simulink model "AcasXuClosedLoop_all_comp"  (Simulink 10.0)
settings: Solver=VariableStepAuto  StartTime=0.0  StopTime=20  RelTol=1e-3
libraries: neural x14040, simulink x2
(part 10 of 534)

== system AcasXuClosedLoop_all_comp/networks/Networks_m_2/AcasXu_1_2/Layer 5 ==
  "a{4} " : Inport  PortDimensions=50
      mask Display: e = 0.06;c1 = [120 230 180]/255;c2 = ([120 230 180]+0)/(256+128);c3 = ([120 230 180]+128)/(256+128);patch([0 1 1 0],[0 0 1 1],c1);patch([0 1 1 1-e 1-e 0+e],[0 0 1 1-e 0+e 0+e],c2);patch([0 0 1 1-e 0+e 0+e],[0 1 1 1-e 1-e 0+e],c3);plot([0 1 1 0 0],[0 0 1 1 0]);
  Delays 1 : Subsystem
      mask Display: e = 0.06;c1 = [120 150 230]/255;c2 = ([120 150 230]+0)/383;c3 = ([120 150 230]+128)/383;patch([0 1 1 0],[0 0 1 1],c1);patch([0 1 1 1-e 1-e 0+e],[0 0 1 1-e 0+e 0+e],c2);patch([0 0 1 1-e 0+e 0+e],[0 1 1 1-e 1-e 0+e],c3);plot([0 1 1 0 0],[0 0 1 1 0]);disp('0');
  "LW{5,4}" : Subsystem
      mask Display: e = 0.06;c1 = [120 150 230]/255;c2 = ([120 150 230]+0)/383;c3 = ([120 150 230]+128)/383;patch([0 1 1 0],[0 0 1 1],c1);patch([0 1 1 1-e 1-e 0+e],[0 0 1 1-e 0+e 0+e],c2);patch([0 0 1 1-e 0+e 0+e],[0 1 1 1-e 1-e 0+e],c3);plot([0 1 1 0 0],[0 0 1 1 0]);disp('W');
  "b{5}" : Constant  Value=[-3.09175000000000022026824808563105762004852294921875;-1.5789699999999999846522769075818359851837158203125;-0.74790400000000001323741116721066646277904510498046875;0.418426000000000020140333845120039768517017364501953125;-0.018132399999999999906652448089516838081181049346923828125;0.7196780000000000399040800402872264385223388671875;0.1770190000000000096758157042131642811000347137451171875;-0.259960000000000024389379404965438880026340484619140625;-2.82767999999999997129407347529195249080657958984375;-0.46265400000000000968469748841016553342342376708984375;-3.398219999999999796358451931155286729335784912109375;1.55956999999999990080823408789001405239105224609375;0.96224100000000001298161578233703039586544036865234375;-2.47121000000000012875034371973015367984771728515625;-0.042217499999999998083755059496979811228811740875244140625;0.29803400000000002112443553414777852594852447509765625;-0.62363299999999999290167806975659914314746856689453125;-0.1538940000000000030144775564622250385582447052001953125;-0.1909220000000000083684170704145799390971660614013671875;-0.435248000000000023756996370138949714601039886474609375;0.45485399999999998055244532224605791270732879638671875;0.197116000000000013425704992187093012034893035888671875;0.02747940000000000104751762819432769902050495147705078125;-1.5378700000000000702726765666739083826541900634765625;1.596940000000000026147972675971686840057373046875;-1.3756299999999999084110413605230860412120819091796875;0.65624899999999997124433548378874547779560089111328125;0.0542021000000000030549784924005507491528987884521484375;-0.6906120000000000036521896618069149553775787353515625;-0.117754999999999998561150960085797123610973358154296875;-0.1625709999999999932907002175852539949119091033935546875;-0.76035200000000002784616981443832628428936004638671875;-0.319209000000000020502710640357690863311290740966796875;-0.465017000000000013670842236024327576160430908203125;0.1303910000000000068975936073911725543439388275146484375;0.0582017999999999979809928163376753218472003936767578125;1.6693700000000000205346850634668953716754913330078125;-0.413046000000000024243718144134618341922760009765625;-0.93940400000000001679012484601116739213466644287109375;-1.9987999999999999101163439263473264873027801513671875;0.44529900000000000037658764995285309851169586181640625;-1.321730000000000071480599217466078698635101318359375;0.44816299999999997805133489237050525844097137451171875;-0.069501800000000002466293835823307745158672332763671875;0.337838000000000027167601501787430606782436370849609375;1.7101599999999999024424823801382444798946380615234375;1.6649700000000000610356210017926059663295745849609375;0.54888499999999995626609461396583355963230133056640625;-0.51785099999999995024069221472018398344516754150390625;0.66518999999999994798827174236066639423370361328125]
      mask Display: e = 0.06;c1 = [120 150 230]/255;c2 = ([120 150 230]+0)/383;c3 = ([120 150 230]+128)/383;patch([0 1 1 0],[0 0 1 1],c1);patch([0 1 1 1-e 1-e 0+e],[0 0 1 1-e 0+e 0+e],c2);patch([0 0 1 1-e 0+e 0+e],[0 1 1 1-e 1-e 0+e],c3);plot([0 1 1 0 0],[0 0 1 1 0]);disp('b');
  netsum : Sum  Inputs=2  SaturateOnIntegerOverflow=on  InputSameDT=on  OutDataTypeStr=Inherit: Same as first input
      mask Display: e = 0.06;c1 = [120 150 230]/255;c2 = ([120 150 230]+0)/383;c3 = ([120 150 230]+128)/383;patch([0 1 1 0],[0 0 1 1],c1);patch([0 1 1 1-e 1-e 0+e],[0 0 1 1-e 0+e 0+e],c2);patch([0 0 1 1-e 0+e 0+e],[0 1 1 1-e 1-e 0+e],c3);plot([0 1 1 0 0],[0 0 1 1 0]);disp('+');
  poslin : neural/Transfer Functions/poslin
      settings: SourceProductName=Deep Learning Toolbox  SourceProductBaseCode=NN
  "a{5}" : Outport  InitialOutput=[0;0;0;0;0;0;0;0;0;0;0;0;0;0;0;0;0;0;0;0;0;0;0;0;0;0;0;0;0;0;0;0;0;0;0;0;0;0;0;0;0;0;0;0;0;0;0;0;0;0]
      mask Display: e = 0.06;c1 = [120 230 180]/255;c2 = ([120 230 180]+0)/(256+128);c3 = ([120 230 180]+128)/(256+128);patch([0 1 1 0],[0 0 1 1],c1);patch([0 1 1 1-e 1-e 0+e],[0 0 1 1-e 0+e 0+e],c2);patch([0 0 1 1-e 0+e 0+e],[0 1 1 1-e 1-e 0+e],c3);plot([0 1 1 0 0],[0 0 1 1 0]);
  wires:
  poslin/1 -> "a{5}"/1
  netsum/1 -> poslin/1
  "b{5}"/1 -> netsum/2
  "LW{5,4}"/1("lz{5,4}") -> netsum/1
  Delays 1/1("pd{5,4}") -> "LW{5,4}"/1("ad{5,4}")
  "a{4} "/1 -> Delays 1/1("p{5}")

== system AcasXuClosedLoop_all_comp/networks/Networks_m_2/AcasXu_1_2/Layer 6 ==
  "a{5} " : Inport  PortDimensions=50
      mask Display: e = 0.06;c1 = [120 230 180]/255;c2 = ([120 230 180]+0)/(256+128);c3 = ([120 230 180]+128)/(256+128);patch([0 1 1 0],[0 0 1 1],c1);patch([0 1 1 1-e 1-e 0+e],[0 0 1 1-e 0+e 0+e],c2);patch([0 0 1 1-e 0+e 0+e],[0 1 1 1-e 1-e 0+e],c3);plot([0 1 1 0 0],[0 0 1 1 0]);
  Delays 1 : Subsystem
      mask Display: e = 0.06;c1 = [120 150 230]/255;c2 = ([120 150 230]+0)/383;c3 = ([120 150 230]+128)/383;patch([0 1 1 0],[0 0 1 1],c1);patch([0 1 1 1-e 1-e 0+e],[0 0 1 1-e 0+e 0+e],c2);patch([0 0 1 1-e 0+e 0+e],[0 1 1 1-e 1-e 0+e],c3);plot([0 1 1 0 0],[0 0 1 1 0]);disp('0');
  "LW{6,5}" : Subsystem
      mask Display: e = 0.06;c1 = [120 150 230]/255;c2 = ([120 150 230]+0)/383;c3 = ([120 150 230]+128)/383;patch([0 1 1 0],[0 0 1 1],c1);patch([0 1 1 1-e 1-e 0+e],[0 0 1 1-e 0+e 0+e],c2);patch([0 0 1 1-e 0+e 0+e],[0 1 1 1-e 1-e 0+e],c3);plot([0 1 1 0 0],[0 0 1 1 0]);disp('W');
  "b{6}" : Constant  Value=[-0.341378000000000014768630762773682363331317901611328125;-1.752289999999999903224079389474354684352874755859375;-0.2081030000000000101945119013180374167859554290771484375;-0.1790799999999999891908686322494759224355220794677734375;-1.8451100000000000278532752417959272861480712890625;0.09219460000000000154951607100883848033845424652099609375;0.19085099999999999287325636032619513571262359619140625;0.1194800000000000028688162956314045004546642303466796875;-2.408889999999999975699438437004573643207550048828125;-4.517640000000000100044417195022106170654296875;-0.15426200000000001022470996758784167468547821044921875;-1.578440000000000065227823142777197062969207763671875;-3.6915800000000000835598257253877818584442138671875;0.91479699999999997128696804793435148894786834716796875;-0.38159799999999999275956952260457910597324371337890625;-1.4166499999999999648281345798750407993793487548828125;0.4794840000000000213020712180878035724163055419921875;0.0837204000000000003733902076419326476752758026123046875;-0.149436999999999986510346161594497971236705780029296875;-0.476080000000000003179678742526448331773281097412109375;-2.065690000000000026147972675971686840057373046875;-0.488171999999999994823696169987670145928859710693359375;-5.1282399999999999096189640113152563571929931640625;-0.326983999999999996877164676334359683096408843994140625;0.029691399999999999737010369926792918704450130462646484375;0.159138000000000001676880856393836438655853271484375;0.169696000000000013496759265763103030622005462646484375;-0.7378299999999999858601995583740063011646270751953125;-0.377545999999999992713384244780172593891620635986328125;0.1332689999999999985735854579615988768637180328369140625;0.1173160000000000036113334545007091946899890899658203125;0.084497400000000000286348722511320374906063079833984375;-5.8619000000000003325340003357268869876861572265625;-1.7486800000000000121502807814977131783962249755859375;1.5344800000000000661515286992653273046016693115234375;-0.42604399999999997827870856781373731791973114013671875;0.1529330000000000133741906438444857485592365264892578125;0.443421000000000009588774219082552008330821990966796875;-0.54626600000000002932409870481933467090129852294921875;-1.263570000000000081996631706715561449527740478515625;0.2061469999999999969109154562829644419252872467041015625;-0.86114699999999999580069243165780790150165557861328125;-0.09318210000000000381437104124415782280266284942626953125;0.23265299999999999869260136620141565799713134765625;0.325915000000000010249578963339445181190967559814453125;1.28146000000000004348521542851813137531280517578125;0.473376999999999992230215184463304467499256134033203125;-0.14630799999999999361222080551669932901859283447265625;0.080574599999999996224886444906587712466716766357421875;-0.1077599999999999946798112659962498582899570465087890625]
      mask Display: e = 0.06;c1 = [120 150 230]/255;c2 = ([120 150 230]+0)/383;c3 = ([120 150 230]+128)/383;patch([0 1 1 0],[0 0 1 1],c1);patch([0 1 1 1-e 1-e 0+e],[0 0 1 1-e 0+e 0+e],c2);patch([0 0 1 1-e 0+e 0+e],[0 1 1 1-e 1-e 0+e],c3);plot([0 1 1 0 0],[0 0 1 1 0]);disp('b');
  netsum : Sum  Inputs=2  SaturateOnIntegerOverflow=on  InputSameDT=on  OutDataTypeStr=Inherit: Same as first input
      mask Display: e = 0.06;c1 = [120 150 230]/255;c2 = ([120 150 230]+0)/383;c3 = ([120 150 230]+128)/383;patch([0 1 1 0],[0 0 1 1],c1);patch([0 1 1 1-e 1-e 0+e],[0 0 1 1-e 0+e 0+e],c2);patch([0 0 1 1-e 0+e 0+e],[0 1 1 1-e 1-e 0+e],c3);plot([0 1 1 0 0],[0 0 1 1 0]);disp('+');
  poslin : neural/Transfer Functions/poslin
      settings: SourceProductName=Deep Learning Toolbox  SourceProductBaseCode=NN
  "a{6}" : Outport  InitialOutput=[0;0;0;0;0;0;0;0;0;0;0;0;0;0;0;0;0;0;0;0;0;0;0;0;0;0;0;0;0;0;0;0;0;0;0;0;0;0;0;0;0;0;0;0;0;0;0;0;0;0]
      mask Display: e = 0.06;c1 = [120 230 180]/255;c2 = ([120 230 180]+0)/(256+128);c3 = ([120 230 180]+128)/(256+128);patch([0 1 1 0],[0 0 1 1],c1);patch([0 1 1 1-e 1-e 0+e],[0 0 1 1-e 0+e 0+e],c2);patch([0 0 1 1-e 0+e 0+e],[0 1 1 1-e 1-e 0+e],c3);plot([0 1 1 0 0],[0 0 1 1 0]);
  wires:
  poslin/1 -> "a{6}"/1
  netsum/1 -> poslin/1
  "b{6}"/1 -> netsum/2
  "LW{6,5}"/1("lz{6,5}") -> netsum/1
  Delays 1/1("pd{6,5}") -> "LW{6,5}"/1("ad{6,5}")
  "a{5} "/1 -> Delays 1/1("p{6}")

== system AcasXuClosedLoop_all_comp/networks/Networks_m_2/AcasXu_1_2/Layer 7 ==
  "a{6} " : Inport  PortDimensions=50
      mask Display: e = 0.06;c1 = [120 230 180]/255;c2 = ([120 230 180]+0)/(256+128);c3 = ([120 230 180]+128)/(256+128);patch([0 1 1 0],[0 0 1 1],c1);patch([0 1 1 1-e 1-e 0+e],[0 0 1 1-e 0+e 0+e],c2);patch([0 0 1 1-e 0+e 0+e],[0 1 1 1-e 1-e 0+e],c3);plot([0 1 1 0 0],[0 0 1 1 0]);
  Delays 1 : Subsystem
      mask Display: e = 0.06;c1 = [120 150 230]/255;c2 = ([120 150 230]+0)/383;c3 = ([120 150 230]+128)/383;patch([0 1 1 0],[0 0 1 1],c1);patch([0 1 1 1-e 1-e 0+e],[0 0 1 1-e 0+e 0+e],c2);patch([0 0 1 1-e 0+e 0+e],[0 1 1 1-e 1-e 0+e],c3);plot([0 1 1 0 0],[0 0 1 1 0]);disp('0');
  "LW{7,6}" : Subsystem
      mask Display: e = 0.06;c1 = [120 150 230]/255;c2 = ([120 150 230]+0)/383;c3 = ([120 150 230]+128)/383;patch([0 1 1 0],[0 0 1 1],c1);patch([0 1 1 1-e 1-e 0+e],[0 0 1 1-e 0+e 0+e],c2);patch([0 0 1 1-e 0+e 0+e],[0 1 1 1-e 1-e 0+e],c3);plot([0 1 1 0 0],[0 0 1 1 0]);disp('W');
  "b{7}" : Constant  Value=[-0.0202845000000000004469757897140880231745541095733642578125;-0.019308499999999999274802320314847747795283794403076171875;-0.019157000000000000416999768049208796583116054534912109375;-0.018912800000000000444355663375972653739154338836669921875;-0.0189197000000000010999645638776200939901173114776611328125]
      mask Display: e = 0.06;c1 = [120 150 230]/255;c2 = ([120 150 230]+0)/383;c3 = ([120 150 230]+128)/383;patch([0 1 1 0],[0 0 1 1],c1);patch([0 1 1 1-e 1-e 0+e],[0 0 1 1-e 0+e 0+e],c2);patch([0 0 1 1-e 0+e 0+e],[0 1 1 1-e 1-e 0+e],c3);plot([0 1 1 0 0],[0 0 1 1 0]);disp('b');
  netsum : Sum  Inputs=2  SaturateOnIntegerOverflow=on  InputSameDT=on  OutDataTypeStr=Inherit: Same as first input
      mask Display: e = 0.06;c1 = [120 150 230]/255;c2 = ([120 150 230]+0)/383;c3 = ([120 150 230]+128)/383;patch([0 1 1 0],[0 0 1 1],c1);patch([0 1 1 1-e 1-e 0+e],[0 0 1 1-e 0+e 0+e],c2);patch([0 0 1 1-e 0+e 0+e],[0 1 1 1-e 1-e 0+e],c3);plot([0 1 1 0 0],[0 0 1 1 0]);disp('+');
  purelin : neural/Transfer Functions/purelin
      settings: SourceProductName=Deep Learning Toolbox
  "a{7}" : Outport  InitialOutput=[0;0;0;0;0]
      mask Display: e = 0.06;c1 = [120 230 180]/255;c2 = ([120 230 180]+0)/(256+128);c3 = ([120 230 180]+128)/(256+128);patch([0 1 1 0],[0 0 1 1],c1);patch([0 1 1 1-e 1-e 0+e],[0 0 1 1-e 0+e 0+e],c2);patch([0 0 1 1-e 0+e 0+e],[0 1 1 1-e 1-e 0+e],c3);plot([0 1 1 0 0],[0 0 1 1 0]);
  wires:
  purelin/1 -> "a{7}"/1
  netsum/1 -> purelin/1
  "b{7}"/1 -> netsum/2
  "LW{7,6}"/1("lz{7,6}") -> netsum/1
  Delays 1/1("pd{7,6}") -> "LW{7,6}"/1("ad{7,6}")
  "a{6} "/1 -> Delays 1/1("p{7}")

== system AcasXuClosedLoop_all_comp/networks/Networks_m_2/AcasXu_1_2/Process Input 1 ==
  x : Inport  PortDimensions=5
      mask Display: e = 0.06;c1 = [120 230 180]/255;c2 = ([120 230 180]+0)/(256+128);c3 = ([120 230 180]+128)/(256+128);patch([0 1 1 0],[0 0 1 1],c1);patch([0 1 1 1-e 1-e 0+e],[0 0 1 1-e 0+e 0+e],c2);patch([0 0 1 1-e 0+e 0+e],[0 1 1 1-e 1-e 0+e],c3);plot([0 1 1 0 0],[0 0 1 1 0]);
  p : Outport  PortDimensions=5
      mask Display: e = 0.06;c1 = [120 230 180]/255;c2 = ([120 230 180]+0)/(256+128);c3 = ([120 230 180]+128)/(256+128);patch([0 1 1 0],[0 0 1 1],c1);patch([0 1 1 1-e 1-e 0+e],[0 0 1 1-e 0+e 0+e],c2);patch([0 0 1 1-e 0+e 0+e],[0 1 1 1-e 1-e 0+e],c3);plot([0 1 1 0 0],[0 0 1 1 0]);
  wires:
  x/1 -> p/1

== system AcasXuClosedLoop_all_comp/networks/Networks_m_2/AcasXu_1_2/Process Output 1 ==
  a : Inport  PortDimensions=5
      mask Display: e = 0.06;c1 = [120 230 180]/255;c2 = ([120 230 180]+0)/(256+128);c3 = ([120 230 180]+128)/(256+128);patch([0 1 1 0],[0 0 1 1],c1);patch([0 1 1 1-e 1-e 0+e],[0 0 1 1-e 0+e 0+e],c2);patch([0 0 1 1-e 0+e 0+e],[0 1 1 1-e 1-e 0+e],c3);plot([0 1 1 0 0],[0 0 1 1 0]);
  y : Outport  PortDimensions=5
      mask Display: e = 0.06;c1 = [120 230 180]/255;c2 = ([120 230 180]+0)/(256+128);c3 = ([120 230 180]+128)/(256+128);patch([0 1 1 0],[0 0 1 1],c1);patch([0 1 1 1-e 1-e 0+e],[0 0 1 1-e 0+e 0+e],c2);patch([0 0 1 1-e 0+e 0+e],[0 1 1 1-e 1-e 0+e],c3);plot([0 1 1 0 0],[0 0 1 1 0]);
  wires:
  a/1 -> y/1

== system AcasXuClosedLoop_all_comp/networks/Networks_m_2/AcasXu_2_2/Layer 1 ==
  "p{1}" : Inport  PortDimensions=5
      mask Display: e = 0.06;c1 = [120 230 180]/255;c2 = ([120 230 180]+0)/(256+128);c3 = ([120 230 180]+128)/(256+128);patch([0 1 1 0],[0 0 1 1],c1);patch([0 1 1 1-e 1-e 0+e],[0 0 1 1-e 0+e 0+e],c2);patch([0 0 1 1-e 0+e 0+e],[0 1 1 1-e 1-e 0+e],c3);plot([0 1 1 0 0],[0 0 1 1 0]);
  Delays 1 : Subsystem
      mask Display: e = 0.06;c1 = [120 150 230]/255;c2 = ([120 150 230]+0)/383;c3 = ([120 150 230]+128)/383;patch([0 1 1 0],[0 0 1 1],c1);patch([0 1 1 1-e 1-e 0+e],[0 0 1 1-e 0+e 0+e],c2);patch([0 0 1 1-e 0+e 0+e],[0 1 1 1-e 1-e 0+e],c3);plot([0 1 1 0 0],[0 0 1 1 0]);disp('0');
  "IW{1,1}" : Subsystem
      mask Display: e = 0.06;c1 = [120 150 230]/255;c2 = ([120 150 230]+0)/383;c3 = ([120 150 230]+128)/383;patch([0 1 1 0],[0 0 1 1],c1);patch([0 1 1 1-e 1-e 0+e],[0 0 1 1-e 0+e 0+e],c2);patch([0 0 1 1-e 0+e 0+e],[0 1 1 1-e 1-e 0+e],c3);plot([0 1 1 0 0],[0 0 1 1 0]);disp('W');
  "b{1}" : Constant  Value=[-0.71073200000000003040412366317468695342540740966796875;-0.023406599999999999628386149197467602789402008056640625;-0.1468069999999999930562211147844209335744380950927734375;0.1924019999999999896989066883179475553333759307861328125;0.34060699999999999310063003576942719519138336181640625;0.0814416000000000028791191652999259531497955322265625;-0.21351500000000001033839680530945770442485809326171875;0.127441999999999999726441046732361428439617156982421875;-0.1905419999999999891571178523008711636066436767578125;-0.1620499999999999996003197111349436454474925994873046875;-0.082190499999999999669597627871553413569927215576171875;-0.64436499999999996557420445242314599454402923583984375;-0.53278400000000003533529024934978224337100982666015625;-0.60548100000000004694555855166981928050518035888671875;-0.0231250999999999991285637435112221282906830310821533203125;-0.0289112000000000014809931059289738186635076999664306640625;-0.3672210000000000196251903616939671337604522705078125;-0.277160000000000017461587731304462067782878875732421875;0.246352999999999988656185223590000532567501068115234375;-0.059020099999999998952038282595822238363325595855712890625;-0.59103399999999994829380511873750947415828704833984375;-0.2083589999999999886615142941082012839615345001220703125;-0.67096599999999995134203345514833927154541015625;0.304474999999999995647925743469386361539363861083984375;-0.1941980000000000095017327339519397355616092681884765625;-0.0832877999999999951707962964064790867269039154052734375;-0.50439299999999998025401737322681583464145660400390625;0.1512320000000000053130833066461491398513317108154296875;0.0917239999999999999769073610877967439591884613037109375;-0.39220500000000002582822844487964175641536712646484375;0.034806999999999997663646666978820576332509517669677734375;-0.0283214000000000000467625937972115934826433658599853515625;0.117306999999999994610533349259640090167522430419921875;-0.45529900000000000925837184695410542190074920654296875;-0.2465559999999999973852027324028313159942626953125;0.11519100000000000172395431263794307596981525421142578125;-0.004594850000000000149469325805284825037233531475067138671875;0.1917580000000000117754694883842603303492069244384765625;-0.765735999999999972231989886495284736156463623046875;-0.056882500000000002504663143554353155195713043212890625;-0.6090430000000000010373923942097462713718414306640625;0.0852509999999999934505723331312765367329120635986328125;-0.3668839999999999879065626373630948364734649658203125;-0.0160115000000000014257484082236260292120277881622314453125;0.1520410000000000094733110245215357281267642974853515625;-0.50538600000000000189714910447946749627590179443359375;0.0280642000000000009507505893679990549571812152862548828125;-0.11279799999999999549071816318246419541537761688232421875;-0.5673850000000000282085466096759773790836334228515625;-0.2479320000000000134843247678873012773692607879638671875]
      mask Display: e = 0.06;c1 = [120 150 230]/255;c2 = ([120 150 230]+0)/383;c3 = ([120 150 230]+128)/383;patch([0 1 1 0],[0 0 1 1],c1);patch([0 1 1 1-e 1-e 0+e],[0 0 1 1-e 0+e 0+e],c2);patch([0 0 1 1-e 0+e 0+e],[0 1 1 1-e 1-e 0+e],c3);plot([0 1 1 0 0],[0 0 1 1 0]);disp('b');
  netsum : Sum  Inputs=2  SaturateOnIntegerOverflow=on  InputSameDT=on  OutDataTypeStr=Inherit: Same as first input
      mask Display: e = 0.06;c1 = [120 150 230]/255;c2 = ([120 150 230]+0)/383;c3 = ([120 150 230]+128)/383;patch([0 1 1 0],[0 0 1 1],c1);patch([0 1 1 1-e 1-e 0+e],[0 0 1 1-e 0+e 0+e],c2);patch([0 0 1 1-e 0+e 0+e],[0 1 1 1-e 1-e 0+e],c3);plot([0 1 1 0 0],[0 0 1 1 0]);disp('+');
  poslin : neural/Transfer Functions/poslin
      settings: SourceProductName=Deep Learning Toolbox  SourceProductBaseCode=NN
  "a{1}" : Outport  InitialOutput=[0;0;0;0;0;0;0;0;0;0;0;0;0;0;0;0;0;0;0;0;0;0;0;0;0;0;0;0;0;0;0;0;0;0;0;0;0;0;0;0;0;0;0;0;0;0;0;0;0;0]
      mask Display: e = 0.06;c1 = [120 230 180]/255;c2 = ([120 230 180]+0)/(256+128);c3 = ([120 230 180]+128)/(256+128);patch([0 1 1 0],[0 0 1 1],c1);patch([0 1 1 1-e 1-e 0+e],[0 0 1 1-e 0+e 0+e],c2);patch([0 0 1 1-e 0+e 0+e],[0 1 1 1-e 1-e 0+e],c3);plot([0 1 1 0 0],[0 0 1 1 0]);
  wires:
  poslin/1 -> "a{1}"/1
  netsum/1 -> poslin/1
  "b{1}"/1 -> netsum/2
  "IW{1,1}"/1("iz{1,1}") -> netsum/1
  Delays 1/1("pd{1,1}") -> "IW{1,1}"/1("pd{1,1}")
  "p{1}"/1 -> Delays 1/1("p{1}")

== system AcasXuClosedLoop_all_comp/networks/Networks_m_2/AcasXu_2_2/Layer 2 ==
  "a{1} " : Inport  PortDimensions=50
      mask Display: e = 0.06;c1 = [120 230 180]/255;c2 = ([120 230 180]+0)/(256+128);c3 = ([120 230 180]+128)/(256+128);patch([0 1 1 0],[0 0 1 1],c1);patch([0 1 1 1-e 1-e 0+e],[0 0 1 1-e 0+e 0+e],c2);patch([0 0 1 1-e 0+e 0+e],[0 1 1 1-e 1-e 0+e],c3);plot([0 1 1 0 0],[0 0 1 1 0]);
  Delays 1 : Subsystem
      mask Display: e = 0.06;c1 = [120 150 230]/255;c2 = ([120 150 230]+0)/383;c3 = ([120 150 230]+128)/383;patch([0 1 1 0],[0 0 1 1],c1);patch([0 1 1 1-e 1-e 0+e],[0 0 1 1-e 0+e 0+e],c2);patch([0 0 1 1-e 0+e 0+e],[0 1 1 1-e 1-e 0+e],c3);plot([0 1 1 0 0],[0 0 1 1 0]);disp('0');
  "LW{2,1}" : Subsystem
      mask Display: e = 0.06;c1 = [120 150 230]/255;c2 = ([120 150 230]+0)/383;c3 = ([120 150 230]+128)/383;patch([0 1 1 0],[0 0 1 1],c1);patch([0 1 1 1-e 1-e 0+e],[0 0 1 1-e 0+e 0+e],c2);patch([0 0 1 1-e 0+e 0+e],[0 1 1 1-e 1-e 0+e],c3);plot([0 1 1 0 0],[0 0 1 1 0]);disp('W');
  "b{2}" : Constant  Value=[-0.12164899999999999324895583185934810899198055267333984375;-0.27929700000000001747224587234086357057094573974609375;-0.09955039999999999722479770980498869903385639190673828125;-0.88822199999999995601029922909219749271869659423828125;0.8940550000000000441247038907022215425968170166015625;-0.85196600000000000108002495835535228252410888671875;-0.1104360000000000063824501239650999195873737335205078125;-0.198512999999999995015542708642897196114063262939453125;-0.0740760000000000029540814239226165227591991424560546875;-1.153350000000000097344354799133725464344024658203125;-0.63325299999999995481658743301522918045520782470703125;-0.94377900000000003455369324001367203891277313232421875;-0.01792950000000000099209529480503988452255725860595703125;0.040906299999999999605915235179054434411227703094482421875;0.170406000000000001914912672873470000922679901123046875;-0.68770399999999998197353079376625828444957733154296875;0.3030559999999999920561322142020799219608306884765625;0.255921000000000009588774219082552008330821990966796875;-0.99250000000000004884981308350688777863979339599609375;0.1765649999999999997246646898929611779749393463134765625;-0.655877999999999961033836370916105806827545166015625;0.646905999999999981042719809920527040958404541015625;1.4067000000000000614619466432486660778522491455078125;-0.339610000000000022968293933445238508284091949462890625;0.641823000000000032372327041230164468288421630859375;1.604179999999999939319650366087444126605987548828125;0.3667730000000000156745727508678101003170013427734375;-0.84923800000000004839506573262042365968227386474609375;-0.7250959999999999627817715008859522640705108642578125;-0.0085157600000000006901235138911943067796528339385986328125;-0.6992819999999999591722144032246433198451995849609375;-0.11682800000000000129052324382428196258842945098876953125;0.52576699999999998436095438592019490897655487060546875;-0.00648930999999999987004617452157617663033306598663330078125;-0.22376099999999998768629438927746377885341644287109375;-0.37841000000000002412292587905540131032466888427734375;0.519700999999999968537167660542763769626617431640625;0.04915540000000000186997084483664366416633129119873046875;-0.042968399999999996874411323233289294876158237457275390625;-0.63150300000000003652900204542675055563449859619140625;-1.0430900000000000726885218682582490146160125732421875;-0.054936400000000003285283156628793221898376941680908203125;-0.0966757000000000032979841080305050127208232879638671875;-0.08870530000000000081517015360077493824064731597900390625;-0.8591100000000000402877731175976805388927459716796875;-0.61526599999999997958610720161232165992259979248046875;-0.031076499999999999956923346644543926231563091278076171875;-0.945826999999999973312014844850637018680572509765625;-0.91236499999999998156141600702540017664432525634765625;0.391450999999999993406163412146270275115966796875]
      mask Display: e = 0.06;c1 = [120 150 230]/255;c2 = ([120 150 230]+0)/383;c3 = ([120 150 230]+128)/383;patch([0 1 1 0],[0 0 1 1],c1);patch([0 1 1 1-e 1-e 0+e],[0 0 1 1-e 0+e 0+e],c2);patch([0 0 1 1-e 0+e 0+e],[0 1 1 1-e 1-e 0+e],c3);plot([0 1 1 0 0],[0 0 1 1 0]);disp('b');
  netsum : Sum  Inputs=2  SaturateOnIntegerOverflow=on  InputSameDT=on  OutDataTypeStr=Inherit: Same as first input
      mask Display: e = 0.06;c1 = [120 150 230]/255;c2 = ([120 150 230]+0)/383;c3 = ([120 150 230]+128)/383;patch([0 1 1 0],[0 0 1 1],c1);patch([0 1 1 1-e 1-e 0+e],[0 0 1 1-e 0+e 0+e],c2);patch([0 0 1 1-e 0+e 0+e],[0 1 1 1-e 1-e 0+e],c3);plot([0 1 1 0 0],[0 0 1 1 0]);disp('+');
  poslin : neural/Transfer Functions/poslin
      settings: SourceProductName=Deep Learning Toolbox  SourceProductBaseCode=NN
  "a{2}" : Outport  InitialOutput=[0;0;0;0;0;0;0;0;0;0;0;0;0;0;0;0;0;0;0;0;0;0;0;0;0;0;0;0;0;0;0;0;0;0;0;0;0;0;0;0;0;0;0;0;0;0;0;0;0;0]
      mask Display: e = 0.06;c1 = [120 230 180]/255;c2 = ([120 230 180]+0)/(256+128);c3 = ([120 230 180]+128)/(256+128);patch([0 1 1 0],[0 0 1 1],c1);patch([0 1 1 1-e 1-e 0+e],[0 0 1 1-e 0+e 0+e],c2);patch([0 0 1 1-e 0+e 0+e],[0 1 1 1-e 1-e 0+e],c3);plot([0 1 1 0 0],[0 0 1 1 0]);
  wires:
  poslin/1 -> "a{2}"/1
  netsum/1 -> poslin/1
  "b{2}"/1 -> netsum/2
  "LW{2,1}"/1("lz{2,1}") -> netsum/1
  Delays 1/1("pd{2,1}") -> "LW{2,1}"/1("ad{2,1}")
  "a{1} "/1 -> Delays 1/1("p{2}")

== system AcasXuClosedLoop_all_comp/networks/Networks_m_2/AcasXu_2_2/Layer 3 ==
  "a{2} " : Inport  PortDimensions=50
      mask Display: e = 0.06;c1 = [120 230 180]/255;c2 = ([120 230 180]+0)/(256+128);c3 = ([120 230 180]+128)/(256+128);patch([0 1 1 0],[0 0 1 1],c1);patch([0 1 1 1-e 1-e 0+e],[0 0 1 1-e 0+e 0+e],c2);patch([0 0 1 1-e 0+e 0+e],[0 1 1 1-e 1-e 0+e],c3);plot([0 1 1 0 0],[0 0 1 1 0]);
  Delays 1 : Subsystem
      mask Display: e = 0.06;c1 = [120 150 230]/255;c2 = ([120 150 230]+0)/383;c3 = ([120 150 230]+128)/383;patch([0 1 1 0],[0 0 1 1],c1);patch([0 1 1 1-e 1-e 0+e],[0 0 1 1-e 0+e 0+e],c2);patch([0 0 1 1-e 0+e 0+e],[0 1 1 1-e 1-e 0+e],c3);plot([0 1 1 0 0],[0 0 1 1 0]);disp('0');
  "LW{3,2}" : Subsystem
      mask Display: e = 0.06;c1 = [120 150 230]/255;c2 = ([120 150 230]+0)/383;c3 = ([120 150 230]+128)/383;patch([0 1 1 0],[0 0 1 1],c1);patch([0 1 1 1-e 1-e 0+e],[0 0 1 1-e 0+e 0+e],c2);patch([0 0 1 1-e 0+e 0+e],[0 1 1 1-e 1-e 0+e],c3);plot([0 1 1 0 0],[0 0 1 1 0]);disp('W');
  "b{3}" : Constant  Value=[-0.264120999999999994667376768120448105037212371826171875;1.019439999999999901802993917954154312610626220703125;1.366330000000000044479975258582271635532379150390625;0.400241999999999986670218277140520513057708740234375;0.920061000000000017706724975141696631908416748046875;2.51973000000000002529532139305956661701202392578125;-0.1667039999999999910773595956925419159233570098876953125;-1.8284599999999999742072986919083632528781890869140625;0.56852300000000000057553961596568115055561065673828125;0.195977000000000012303047469686134718358516693115234375;-0.0461897000000000002017941369558684527873992919921875;0.063801099999999999479172174687846563756465911865234375;-0.36269899999999999362643166023190133273601531982421875;-0.45260299999999997755395497733843512833118438720703125;0.7419350000000000111555209514335729181766510009765625;-0.78901100000000001788436065908172167837619781494140625;-0.11247400000000000452882176205093855969607830047607421875;-0.0411870000000000013873346915715956129133701324462890625;0.424572000000000004948930154569097794592380523681640625;-0.1323910000000000086739504467914230190217494964599609375;-0.70211999999999996635580146175925619900226593017578125;-0.155287000000000008359535286217578686773777008056640625;0.00733787999999999974998221574651324772275984287261962890625;-0.58643599999999995731059243553318083286285400390625;-0.07315449999999999730615485304952017031610012054443359375;0.472536000000000011578293879210832528769969940185546875;-1.15599000000000007304379323613829910755157470703125;-0.606435999999999975074160829535685479640960693359375;1.2991299999999998959765434847213327884674072265625;0.80531900000000000705568936609779484570026397705078125;2.35156000000000009464429240324534475803375244140625;0.82914399999999999213429191513569094240665435791015625;-0.48423300000000002452082981108105741441249847412109375;-0.90704399999999996140331859351135790348052978515625;-0.040279500000000002801758824944045045413076877593994140625;0.049025300000000000821831491748525877483189105987548828125;-0.32336500000000001353583911622990854084491729736328125;0.198807000000000011485923323562019504606723785400390625;0.01143079999999999964155339426952195935882627964019775390625;-1.39806000000000008043343768804334104061126708984375;-1.37755999999999989569232639041729271411895751953125;-0.27245200000000002749089844655827619135379791259765625;-0.574049000000000031462832339457236230373382568359375;-1.0890999999999999570121644865139387547969818115234375;0.350509000000000014996004438216914422810077667236328125;-1.3330200000000000937205868467572145164012908935546875;1.199829999999999952109419609769247472286224365234375;-1.9733899999999999774757952764048241078853607177734375;1.0203800000000000647304432277451269328594207763671875;1.902930000000000010373923942097462713718414306640625]
      mask Display: e = 0.06;c1 = [120 150 230]/255;c2 = ([120 150 230]+0)/383;c3 = ([120 150 230]+128)/383;patch([0 1 1 0],[0 0 1 1],c1);patch([0 1 1 1-e 1-e 0+e],[0 0 1 1-e 0+e 0+e],c2);patch([0 0 1 1-e 0+e 0+e],[0 1 1 1-e 1-e 0+e],c3);plot([0 1 1 0 0],[0 0 1 1 0]);disp('b');
  netsum : Sum  Inputs=2  SaturateOnIntegerOverflow=on  InputSameDT=on  OutDataTypeStr=Inherit: Same as first input
      mask Display: e = 0.06;c1 = [120 150 230]/255;c2 = ([120 150 230]+0)/383;c3 = ([120 150 230]+128)/383;patch([0 1 1 0],[0 0 1 1],c1);patch([0 1 1 1-e 1-e 0+e],[0 0 1 1-e 0+e 0+e],c2);patch([0 0 1 1-e 0+e 0+e],[0 1 1 1-e 1-e 0+e],c3);plot([0 1 1 0 0],[0 0 1 1 0]);disp('+');
  poslin : neural/Transfer Functions/poslin
      settings: SourceProductName=Deep Learning Toolbox  SourceProductBaseCode=NN
  "a{3}" : Outport  InitialOutput=[0;0;0;0;0;0;0;0;0;0;0;0;0;0;0;0;0;0;0;0;0;0;0;0;0;0;0;0;0;0;0;0;0;0;0;0;0;0;0;0;0;0;0;0;0;0;0;0;0;0]
      mask Display: e = 0.06;c1 = [120 230 180]/255;c2 = ([120 230 180]+0)/(256+128);c3 = ([120 230 180]+128)/(256+128);patch([0 1 1 0],[0 0 1 1],c1);patch([0 1 1 1-e 1-e 0+e],[0 0 1 1-e 0+e 0+e],c2);patch([0 0 1 1-e 0+e 0+e],[0 1 1 1-e 1-e 0+e],c3);plot([0 1 1 0 0],[0 0 1 1 0]);
  wires:
  poslin/1 -> "a{3}"/1
  netsum/1 -> poslin/1
  "b{3}"/1 -> netsum/2
  "LW{3,2}"/1("lz{3,2}") -> netsum/1
  Delays 1/1("pd{3,2}") -> "LW{3,2}"/1("ad{3,2}")
  "a{2} "/1 -> Delays 1/1("p{3}")

== system AcasXuClosedLoop_all_comp/networks/Networks_m_2/AcasXu_2_2/Layer 4 ==
  "a{3} " : Inport  PortDimensions=50
      mask Display: e = 0.06;c1 = [120 230 180]/255;c2 = ([120 230 180]+0)/(256+128);c3 = ([120 230 180]+128)/(256+128);patch([0 1 1 0],[0 0 1 1],c1);patch([0 1 1 1-e 1-e 0+e],[0 0 1 1-e 0+e 0+e],c2);patch([0 0 1 1-e 0+e 0+e],[0 1 1 1-e 1-e 0+e],c3);plot([0 1 1 0 0],[0 0 1 1 0]);
  Delays 1 : Subsystem
      mask Display: e = 0.06;c1 = [120 150 230]/255;c2 = ([120 150 230]+0)/383;c3 = ([120 150 230]+128)/383;patch([0 1 1 0],[0 0 1 1],c1);patch([0 1 1 1-e 1-e 0+e],[0 0 1 1-e 0+e 0+e],c2);patch([0 0 1 1-e 0+e 0+e],[0 1 1 1-e 1-e 0+e],c3);plot([0 1 1 0 0],[0 0 1 1 0]);disp('0');
  "LW{4,3}" : Subsystem
      mask Display: e = 0.06;c1 = [120 150 230]/255;c2 = ([120 150 230]+0)/383;c3 = ([120 150 230]+128)/383;patch([0 1 1 0],[0 0 1 1],c1);patch([0 1 1 1-e 1-e 0+e],[0 0 1 1-e 0+e 0+e],c2);patch([0 0 1 1-e 0+e 0+e],[0 1 1 1-e 1-e 0+e],c3);plot([0 1 1 0 0],[0 0 1 1 0]);disp('W');
  "b{4}" : Constant  Value=[-0.44458100000000000395772303818375803530216217041015625;-0.6136500000000000287769807982840575277805328369140625;1.539800000000000057553961596568115055561065673828125;0.03728309999999999957420726559575996361672878265380859375;1.015309999999999934772176857222802937030792236328125;0.5130829999999999557758201262913644313812255859375;-0.388458000000000025497826072751195169985294342041015625;0.7490010000000000278674860965111292898654937744140625;-0.83132099999999997663735484820790588855743408203125;1.306810000000000027142732506035827100276947021484375;-0.71396599999999998953370550225372426211833953857421875;-1.0359100000000001084998757505672983825206756591796875;0.6877590000000000092228447101661004126071929931640625;-1.599320000000000074891204349114559590816497802734375;-1.0105299999999999283062379618058912456035614013671875;-0.00995919999999999965012431601962816785089671611785888671875;0.64485800000000004228439820508356206119060516357421875;-0.824443000000000036919800550094805657863616943359375;-0.35442499999999999005240169935859739780426025390625;1.27400000000000002131628207280300557613372802734375;-0.26172899999999998943422951924731023609638214111328125;0.1671620000000000050288662123421090655028820037841796875;-0.0224095999999999982710274792907512164674699306488037109375;-0.64144400000000001416111672369879670441150665283203125;0.498035999999999978715692350306198932230472564697265625;-2.885639999999999982804865794605575501918792724609375;-0.60279599999999999848654397283098660409450531005859375;0.8584739999999999593427446598070673644542694091796875;-2.10681999999999991501908880309201776981353759765625;0.86424500000000004096278871656977571547031402587890625;0.631205000000000016058265828178264200687408447265625;1.1473800000000000665068000671453773975372314453125;0.88716799999999995662847140920348465442657470703125;-0.297086000000000016729728713471558876335620880126953125;-0.15964400000000000812150346973794512450695037841796875;-0.1458419999999999994155785998373175971210002899169921875;0.2073649999999999937738692779021221213042736053466796875;-1.4773099999999999010213969086180441081523895263671875;1.3907199999999999562305674771778285503387451171875;-1.79671000000000002927436071331612765789031982421875;-1.353730000000000099902308647870086133480072021484375;0.97067000000000003279154725532862357795238494873046875;0.39004600000000000381561449103173799812793731689453125;-1.051250000000000017763568394002504646778106689453125;0.52064100000000002044231450781808234751224517822265625;-0.4126469999999999860307298149564303457736968994140625;1.4720299999999999496225200346088968217372894287109375;-0.187323999999999990517807191281463019549846649169921875;-0.853366999999999986670218277140520513057708740234375;-1.163699999999999956656893118633888661861419677734375]
      mask Display: e = 0.06;c1 = [120 150 230]/255;c2 = ([120 150 230]+0)/383;c3 = ([120 150 230]+128)/383;patch([0 1 1 0],[0 0 1 1],c1);patch([0 1 1 1-e 1-e 0+e],[0 0 1 1-e 0+e 0+e],c2);patch([0 0 1 1-e 0+e 0+e],[0 1 1 1-e 1-e 0+e],c3);plot([0 1 1 0 0],[0 0 1 1 0]);disp('b');
  netsum : Sum  Inputs=2  SaturateOnIntegerOverflow=on  InputSameDT=on  OutDataTypeStr=Inherit: Same as first input
      mask Display: e = 0.06;c1 = [120 150 230]/255;c2 = ([120 150 230]+0)/383;c3 = ([120 150 230]+128)/383;patch([0 1 1 0],[0 0 1 1],c1);patch([0 1 1 1-e 1-e 0+e],[0 0 1 1-e 0+e 0+e],c2);patch([0 0 1 1-e 0+e 0+e],[0 1 1 1-e 1-e 0+e],c3);plot([0 1 1 0 0],[0 0 1 1 0]);disp('+');
  poslin : neural/Transfer Functions/poslin
      settings: SourceProductName=Deep Learning Toolbox  SourceProductBaseCode=NN
  "a{4}" : Outport  InitialOutput=[0;0;0;0;0;0;0;0;0;0;0;0;0;0;0;0;0;0;0;0;0;0;0;0;0;0;0;0;0;0;0;0;0;0;0;0;0;0;0;0;0;0;0;0;0;0;0;0;0;0]
      mask Display: e = 0.06;c1 = [120 230 180]/255;c2 = ([120 230 180]+0)/(256+128);c3 = ([120 230 180]+128)/(256+128);patch([0 1 1 0],[0 0 1 1],c1);patch([0 1 1 1-e 1-e 0+e],[0 0 1 1-e 0+e 0+e],c2);patch([0 0 1 1-e 0+e 0+e],[0 1 1 1-e 1-e 0+e],c3);plot([0 1 1 0 0],[0 0 1 1 0]);
  wires:
  poslin/1 -> "a{4}"/1
  netsum/1 -> poslin/1
  "b{4}"/1 -> netsum/2
  "LW{4,3}"/1("lz{4,3}") -> netsum/1
  Delays 1/1("pd{4,3}") -> "LW{4,3}"/1("ad{4,3}")
  "a{3} "/1 -> Delays 1/1("p{4}")

== system AcasXuClosedLoop_all_comp/networks/Networks_m_2/AcasXu_2_2/Layer 5 ==
  "a{4} " : Inport  PortDimensions=50
      mask Display: e = 0.06;c1 = [120 230 180]/255;c2 = ([120 230 180]+0)/(256+128);c3 = ([120 230 180]+128)/(256+128);patch([0 1 1 0],[0 0 1 1],c1);patch([0 1 1 1-e 1-e 0+e],[0 0 1 1-e 0+e 0+e],c2);patch([0 0 1 1-e 0+e 0+e],[0 1 1 1-e 1-e 0+e],c3);plot([0 1 1 0 0],[0 0 1 1 0]);
  Delays 1 : Subsystem
      mask Display: e = 0.06;c1 = [120 150 230]/255;c2 = ([120 150 230]+0)/383;c3 = ([120 150 230]+128)/383;patch([0 1 1 0],[0 0 1 1],c1);patch([0 1 1 1-e 1-e 0+e],[0 0 1 1-e 0+e 0+e],c2);patch([0 0 1 1-e 0+e 0+e],[0 1 1 1-e 1-e 0+e],c3);plot([0 1 1 0 0],[0 0 1 1 0]);disp('0');
  "LW{5,4}" : Subsystem
      mask Display: e = 0.06;c1 = [120 150 230]/255;c2 = ([120 150 230]+0)/383;c3 = ([120 150 230]+128)/383;patch([0 1 1 0],[0 0 1 1],c1);patch([0 1 1 1-e 1-e 0+e],[0 0 1 1-e 0+e 0+e],c2);patch([0 0 1 1-e 0+e 0+e],[0 1 1 1-e 1-e 0+e],c3);plot([0 1 1 0 0],[0 0 1 1 0]);disp('W');
  "b{5}" : Constant  Value=[-0.499987000000000014754419908058480359613895416259765625;0.310634999999999994457766661071218550205230712890625;-0.250103000000000019742429913094383664429187774658203125;-0.2603079999999999838422581888153217732906341552734375;-1.77672000000000007702283255639486014842987060546875;-1.648660000000000014352963262354023754596710205078125;-0.352605000000000001758593271006247960031032562255859375;0.736261999999999972033037920482456684112548828125;-0.94427300000000002899724904636968858540058135986328125;-0.31502799999999997471178403429803438484668731689453125;0.435279000000000027004176672562607564032077789306640625;-1.35583000000000009066525308298878371715545654296875;-0.046693900000000003458477948470317642204463481903076171875;-0.96484199999999997743316271225921809673309326171875;0.64108200000000004070699333169613964855670928955078125;-0.557584999999999997299937604111619293689727783203125;-0.83259000000000005226041821515536867082118988037109375;0.391069999999999973194775293450220488011837005615234375;-0.0337700999999999973422148968893452547490596771240234375;-0.325504000000000015546675058430992066860198974609375;-0.0749506000000000061067595424901810474693775177001953125;-0.6806419999999999692619212510180659592151641845703125;0.50772899999999998588151584044680930674076080322265625;1.343669999999999919992887953412719070911407470703125;-0.07546730000000000104787289956220774911344051361083984375;-0.7545960000000000444941861132974736392498016357421875;0.23977999999999999314326259991503320634365081787109375;-0.1722519999999999884554569007377722300589084625244140625;1.8189100000000000267874611381557770073413848876953125;0.55622300000000002295763579240883700549602508544921875;-0.1694199999999999872724032456972054205834865570068359375;-0.1377400000000000013233858453531865961849689483642578125;0.2651310000000000055564441936439834535121917724609375;-0.971028000000000002245315045001916587352752685546875;0.60082599999999997120170291964313946664333343505859375;-1.61577999999999999403144101961515843868255615234375;-1.306880000000000041637804315541870892047882080078125;1.2227399999999999380406734417192637920379638671875;0.371184000000000013930190334576764144003391265869140625;-1.0368500000000000493827201353269629180431365966796875;0.319371000000000015983658840923453681170940399169921875;0.07291499999999999370725589642461272887885570526123046875;-0.85352200000000000290611978925880976021289825439453125;-0.45541500000000001424638185199000872671604156494140625;-2.29389999999999982804865794605575501918792724609375;-0.709149000000000029331204132176935672760009765625;-1.0632800000000000029132252166164107620716094970703125;-0.368613000000000023970159190866979770362377166748046875;-0.032256600000000003325428821199238882400095462799072265625;-1.191920000000000090523144535836763679981231689453125]
      mask Display: e = 0.06;c1 = [120 150 230]/255;c2 = ([120 150 230]+0)/383;c3 = ([120 150 230]+128)/383;patch([0 1 1 0],[0 0 1 1],c1);patch([0 1 1 1-e 1-e 0+e],[0 0 1 1-e 0+e 0+e],c2);patch([0 0 1 1-e 0+e 0+e],[0 1 1 1-e 1-e 0+e],c3);plot([0 1 1 0 0],[0 0 1 1 0]);disp('b');
  netsum : Sum  Inputs=2  SaturateOnIntegerOverflow=on  InputSameDT=on  OutDataTypeStr=Inherit: Same as first input
      mask Display: e = 0.06;c1 = [120 150 230]/255;c2 = ([120 150 230]+0)/383;c3 = ([120 150 230]+128)/383;patch([0 1 1 0],[0 0 1 1],c1);patch([0 1 1 1-e 1-e 0+e],[0 0 1 1-e 0+e 0+e],c2);patch([0 0 1 1-e 0+e 0+e],[0 1 1 1-e 1-e 0+e],c3);plot([0 1 1 0 0],[0 0 1 1 0]);disp('+');
  poslin : neural/Transfer Functions/poslin
      settings: SourceProductName=Deep Learning Toolbox  SourceProductBaseCode=NN
  "a{5}" : Outport  InitialOutput=[0;0;0;0;0;0;0;0;0;0;0;0;0;0;0;0;0;0;0;0;0;0;0;0;0;0;0;0;0;0;0;0;0;0;0;0;0;0;0;0;0;0;0;0;0;0;0;0;0;0]
      mask Display: e = 0.06;c1 = [120 230 180]/255;c2 = ([120 230 180]+0)/(256+128);c3 = ([120 230 180]+128)/(256+128);patch([0 1 1 0],[0 0 1 1],c1);patch([0 1 1 1-e 1-e 0+e],[0 0 1 1-e 0+e 0+e],c2);patch([0 0 1 1-e 0+e 0+e],[0 1 1 1-e 1-e 0+e],c3);plot([0 1 1 0 0],[0 0 1 1 0]);
  wires:
  poslin/1 -> "a{5}"/1
  netsum/1 -> poslin/1
  "b{5}"/1 -> netsum/2
  "LW{5,4}"/1("lz{5,4}") -> netsum/1
  Delays 1/1("pd{5,4}") -> "LW{5,4}"/1("ad{5,4}")
  "a{4} "/1 -> Delays 1/1("p{5}")

== system AcasXuClosedLoop_all_comp/networks/Networks_m_2/AcasXu_2_2/Layer 6 ==
  "a{5} " : Inport  PortDimensions=50
      mask Display: e = 0.06;c1 = [120 230 180]/255;c2 = ([120 230 180]+0)/(256+128);c3 = ([120 230 180]+128)/(256+128);patch([0 1 1 0],[0 0 1 1],c1);patch([0 1 1 1-e 1-e 0+e],[0 0 1 1-e 0+e 0+e],c2);patch([0 0 1 1-e 0+e 0+e],[0 1 1 1-e 1-e 0+e],c3);plot([0 1 1 0 0],[0 0 1 1 0]);
  Delays 1 : Subsystem
      mask Display: e = 0.06;c1 = [120 150 230]/255;c2 = ([120 150 230]+0)/383;c3 = ([120 150 230]+128)/383;patch([0 1 1 0],[0 0 1 1],c1);patch([0 1 1 1-e 1-e 0+e],[0 0 1 1-e 0+e 0+e],c2);patch([0 0 1 1-e 0+e 0+e],[0 1 1 1-e 1-e 0+e],c3);plot([0 1 1 0 0],[0 0 1 1 0]);disp('0');
  "LW{6,5}" : Subsystem
      mask Display: e = 0.06;c1 = [120 150 230]/255;c2 = ([120 150 230]+0)/383;c3 = ([120 150 230]+128)/383;patch([0 1 1 0],[0 0 1 1],c1);patch([0 1 1 1-e 1-e 0+e],[0 0 1 1-e 0+e 0+e],c2);patch([0 0 1 1-e 0+e 0+e],[0 1 1 1-e 1-e 0+e],c3);plot([0 1 1 0 0],[0 0 1 1 0]);disp('W');
  "b{6}" : Constant  Value=[0.2200249999999999983568699235547683201730251312255859375;-0.0478951999999999988855137189602828584611415863037109375;-0.58017700000000005378097966968198306858539581298828125;-0.17723400000000000265032440438517369329929351806640625;-0.57339099999999998402699930011294782161712646484375;-0.364536000000000026677327014112961478531360626220703125;-0.3008830000000000115534248834592290222644805908203125;-1.03763000000000005229594535194337368011474609375;-0.1310099999999999875743839083952479995787143707275390625;-0.3327240000000000197388771994155831634998321533203125;-0.1416469999999999951345586168827139772474765777587890625;-0.1054110000000000046949111265348619781434535980224609375;-0.361391999999999991022292533671134151518344879150390625;-1.0937099999999999599964439767063595354557037353515625;-0.0300049000000000010146550266654230654239654541015625;-0.1162360000000000059827698351000435650348663330078125;-0.050227399999999998436717163485809578560292720794677734375;0.07423839999999999605950762315842439420521259307861328125;-0.4079450000000000020605739337042905390262603759765625;-0.265705000000000024495960815329453907907009124755859375;0.038778600000000003344968746432641637511551380157470703125;-1.0891900000000001025313167701824568212032318115234375;-0.26776400000000000201083594220108352601528167724609375;-1.13712999999999997413624441833235323429107666015625;-0.4884720000000000172946101884008385241031646728515625;0.238605000000000011528555887707625515758991241455078125;0.04211410000000000153352885945423622615635395050048828125;-0.1233910000000000006803446694902959279716014862060546875;-0.531360999999999972231989886495284736156463623046875;-0.1518400000000000027444713168733869679272174835205078125;-0.0726108000000000031182167958831996656954288482666015625;0.13446700000000000319033688356284983456134796142578125;0.04289479999999999682014362178961164318025112152099609375;0.1253640000000000032098768087962525896728038787841796875;0.16803000000000001268318783331778831779956817626953125;-0.544667000000000012249756764504127204418182373046875;-0.4016640000000000210178541237837634980678558349609375;0.08711829999999999574189502027365961112082004547119140625;-0.0340924999999999978061993033406906761229038238525390625;0.15007999999999999118927007657475769519805908203125;0.213195999999999996621369291460723616182804107666015625;0.2033409999999999939745976007543504238128662109375;-0.280411000000000021348256495912210084497928619384765625;-0.12446400000000000518607379262903123162686824798583984375;-0.06340120000000000477502481999181327410042285919189453125;-0.9868230000000000057269744502264074981212615966796875;-0.3010990000000000055280224842135794460773468017578125;-0.1292310000000000125286447882899665273725986480712890625;-0.53115400000000001501376800661091692745685577392578125;-0.022138299999999999645439174855710007250308990478515625]
      mask Display: e = 0.06;c1 = [120 150 230]/255;c2 = ([120 150 230]+0)/383;c3 = ([120 150 230]+128)/383;patch([0 1 1 0],[0 0 1 1],c1);patch([0 1 1 1-e 1-e 0+e],[0 0 1 1-e 0+e 0+e],c2);patch([0 0 1 1-e 0+e 0+e],[0 1 1 1-e 1-e 0+e],c3);plot([0 1 1 0 0],[0 0 1 1 0]);disp('b');
  netsum : Sum  Inputs=2  SaturateOnIntegerOverflow=on  InputSameDT=on  OutDataTypeStr=Inherit: Same as first input
      mask Display: e = 0.06;c1 = [120 150 230]/255;c2 = ([120 150 230]+0)/383;c3 = ([120 150 230]+128)/383;patch([0 1 1 0],[0 0 1 1],c1);patch([0 1 1 1-e 1-e 0+e],[0 0 1 1-e 0+e 0+e],c2);patch([0 0 1 1-e 0+e 0+e],[0 1 1 1-e 1-e 0+e],c3);plot([0 1 1 0 0],[0 0 1 1 0]);disp('+');
  poslin : neural/Transfer Functions/poslin
      settings: SourceProductName=Deep Learning Toolbox  SourceProductBaseCode=NN
  "a{6}" : Outport  InitialOutput=[0;0;0;0;0;0;0;0;0;0;0;0;0;0;0;0;0;0;0;0;0;0;0;0;0;0;0;0;0;0;0;0;0;0;0;0;0;0;0;0;0;0;0;0;0;0;0;0;0;0]
      mask Display: e = 0.06;c1 = [120 230 180]/255;c2 = ([120 230 180]+0)/(256+128);c3 = ([120 230 180]+128)/(256+128);patch([0 1 1 0],[0 0 1 1],c1);patch([0 1 1 1-e 1-e 0+e],[0 0 1 1-e 0+e 0+e],c2);patch([0 0 1 1-e 0+e 0+e],[0 1 1 1-e 1-e 0+e],c3);plot([0 1 1 0 0],[0 0 1 1 0]);
  wires:
  poslin/1 -> "a{6}"/1
  netsum/1 -> poslin/1
  "b{6}"/1 -> netsum/2
  "LW{6,5}"/1("lz{6,5}") -> netsum/1
  Delays 1/1("pd{6,5}") -> "LW{6,5}"/1("ad{6,5}")
  "a{5} "/1 -> Delays 1/1("p{6}")

== system AcasXuClosedLoop_all_comp/networks/Networks_m_2/AcasXu_2_2/Layer 7 ==
  "a{6} " : Inport  PortDimensions=50
      mask Display: e = 0.06;c1 = [120 230 180]/255;c2 = ([120 230 180]+0)/(256+128);c3 = ([120 230 180]+128)/(256+128);patch([0 1 1 0],[0 0 1 1],c1);patch([0 1 1 1-e 1-e 0+e],[0 0 1 1-e 0+e 0+e],c2);patch([0 0 1 1-e 0+e 0+e],[0 1 1 1-e 1-e 0+e],c3);plot([0 1 1 0 0],[0 0 1 1 0]);
  Delays 1 : Subsystem
      mask Display: e = 0.06;c1 = [120 150 230]/255;c2 = ([120 150 230]+0)/383;c3 = ([120 150 230]+128)/383;patch([0 1 1 0],[0 0 1 1],c1);patch([0 1 1 1-e 1-e 0+e],[0 0 1 1-e 0+e 0+e],c2);patch([0 0 1 1-e 0+e 0+e],[0 1 1 1-e 1-e 0+e],c3);plot([0 1 1 0 0],[0 0 1 1 0]);disp('0');
  "LW{7,6}" : Subsystem
      mask Display: e = 0.06;c1 = [120 150 230]/255;c2 = ([120 150 230]+0)/383;c3 = ([120 150 230]+128)/383;patch([0 1 1 0],[0 0 1 1],c1);patch([0 1 1 1-e 1-e 0+e],[0 0 1 1-e 0+e 0+e],c2);patch([0 0 1 1-e 0+e 0+e],[0 1 1 1-e 1-e 0+e],c3);plot([0 1 1 0 0],[0 0 1 1 0]);disp('W');
  "b{7}" : Constant  Value=[-0.0210871000000000009821921054253834881819784641265869140625;-0.0190332000000000001183053655040566809475421905517578125;0.018937900000000000566569013926709885708987712860107421875;-0.0184082999999999989693133528589896741323173046112060546875;0.018053500000000000103028696685214526951313018798828125]
      mask Display: e = 0.06;c1 = [120 150 230]/255;c2 = ([120 150 230]+0)/383;c3 = ([120 150 230]+128)/383;patch([0 1 1 0],[0 0 1 1],c1);patch([0 1 1 1-e 1-e 0+e],[0 0 1 1-e 0+e 0+e],c2);patch([0 0 1 1-e 0+e 0+e],[0 1 1 1-e 1-e 0+e],c3);plot([0 1 1 0 0],[0 0 1 1 0]);disp('b');
  netsum : Sum  Inputs=2  SaturateOnIntegerOverflow=on  InputSameDT=on  OutDataTypeStr=Inherit: Same as first input
      mask Display: e = 0.06;c1 = [120 150 230]/255;c2 = ([120 150 230]+0)/383;c3 = ([120 150 230]+128)/383;patch([0 1 1 0],[0 0 1 1],c1);patch([0 1 1 1-e 1-e 0+e],[0 0 1 1-e 0+e 0+e],c2);patch([0 0 1 1-e 0+e 0+e],[0 1 1 1-e 1-e 0+e],c3);plot([0 1 1 0 0],[0 0 1 1 0]);disp('+');
  purelin : neural/Transfer Functions/purelin
      settings: SourceProductName=Deep Learning Toolbox
  "a{7}" : Outport  InitialOutput=[0;0;0;0;0]
      mask Display: e = 0.06;c1 = [120 230 180]/255;c2 = ([120 230 180]+0)/(256+128);c3 = ([120 230 180]+128)/(256+128);patch([0 1 1 0],[0 0 1 1],c1);patch([0 1 1 1-e 1-e 0+e],[0 0 1 1-e 0+e 0+e],c2);patch([0 0 1 1-e 0+e 0+e],[0 1 1 1-e 1-e 0+e],c3);plot([0 1 1 0 0],[0 0 1 1 0]);
  wires:
  purelin/1 -> "a{7}"/1
  netsum/1 -> purelin/1
  "b{7}"/1 -> netsum/2
  "LW{7,6}"/1("lz{7,6}") -> netsum/1
  Delays 1/1("pd{7,6}") -> "LW{7,6}"/1("ad{7,6}")
  "a{6} "/1 -> Delays 1/1("p{7}")

== system AcasXuClosedLoop_all_comp/networks/Networks_m_2/AcasXu_2_2/Process Input 1 ==
  x : Inport  PortDimensions=5
      mask Display: e = 0.06;c1 = [120 230 180]/255;c2 = ([120 230 180]+0)/(256+128);c3 = ([120 230 180]+128)/(256+128);patch([0 1 1 0],[0 0 1 1],c1);patch([0 1 1 1-e 1-e 0+e],[0 0 1 1-e 0+e 0+e],c2);patch([0 0 1 1-e 0+e 0+e],[0 1 1 1-e 1-e 0+e],c3);plot([0 1 1 0 0],[0 0 1 1 0]);
  p : Outport  PortDimensions=5
      mask Display: e = 0.06;c1 = [120 230 180]/255;c2 = ([120 230 180]+0)/(256+128);c3 = ([120 230 180]+128)/(256+128);patch([0 1 1 0],[0 0 1 1],c1);patch([0 1 1 1-e 1-e 0+e],[0 0 1 1-e 0+e 0+e],c2);patch([0 0 1 1-e 0+e 0+e],[0 1 1 1-e 1-e 0+e],c3);plot([0 1 1 0 0],[0 0 1 1 0]);
  wires:
  x/1 -> p/1
